simulink model "HvacActrPosnReqFrnt"  (R2022b)
settings: Solver=FixedStepDiscrete  StartTime=0.0  StopTime=10.0  FixedStep=0.2  RelTol=1e-3
(part 1 of 2)
settings Model: Creator=blue.ge  LastModifiedBy=blue.ge
settings DataIOCC: SaveFormat=StructureWithTime
settings OptimizationCC: DefaultParameterBehavior=Inlined  EnhancedBackFolding=on  PassReuseOutputArgsAs=Individual arguments  ZeroExternalMemoryAtStartup=off  ZeroInternalMemoryAtStartup=off  OptimizeBlockOrder=speed  DifferentSizesBufferReuse=on  OptimizationCustomize=off  EfficientTunableParamExpr=on
settings DebuggingCC: ParameterTunabilityLossMsg=error
settings ModelReferenceCC: ModelReferenceNumInstancesAllowed=Single
settings SFSimCC: MATLABDynamicMemAlloc=off
settings RTWCC: Description=AUTOSAR  SystemTargetFile=autosar.tlc  GenCodeOnly=on  TemplateMakefile=ert_default_tmf
settings CodeAppCC: ForceParamTrailComments=on  IgnoreCustomStorageClasses=off  OperatorAnnotations=on  SimulinkDataObjDesc=on  SFDataObjDesc=on  InsertBlockDesc=on
settings STFCustomTargetCC: TargetLangStandard=C99 (ISO)  MultiwordLength=256  ParMdlRefBuildCompliant=on  CompOptLevelCompliant=on  IncludeMdlTerminateFcn=off  CombineOutputUpdateFcns=on  SuppressErrorStatus=on  ERTCustomFileBanners=on  SupportNonFinite=off  SupportComplex=off  SupportContinuousTime=off  SupportNonInlinedSFcns=off  RemoveResetFunc=on  MATLABClassNameForMDSCustomization=Simulink.SoftwareTarget.ERTCustomization  AutosarCompliant=on  UseToolchainInfoCompliant=on  LookupTableObjectStructAxisOrder=2,1,3,4,...  LUTObjectStructOrderEvenSpacing=Size,Table,Breakpoints  SystemTargetFile=autosar.tlc
settings ERTTargetCC: TargetLangStandard=C99 (ISO)  ConcurrentExecutionCompliant=off  IncludeMdlTerminateFcn=off  SuppressErrorStatus=on  SupportNonFinite=off  SupportComplex=off  ModelStepFunctionPrototypeControlCompliant=off  CPPClassGenCompliant=off  AutosarCompliant=on  LookupTableObjectStructAxisOrder=2,1,3,4,...  LUTObjectStructOrderEvenSpacing=Size,Table,Breakpoints  InlinedParameterPlacement=NonHierarchical  ERTCustomFileTemplate=example_file_process.tlc

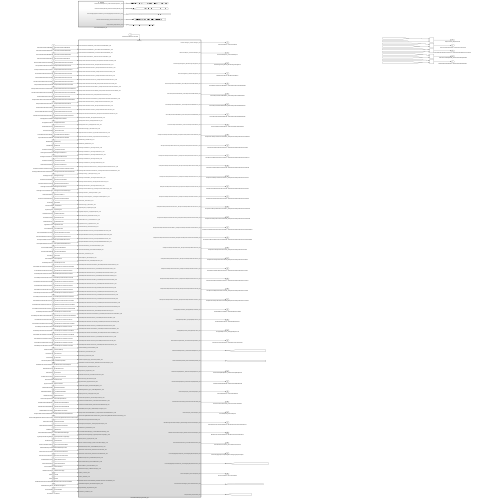
[thumbnail: Simulink's stored preview of the top-level diagram]
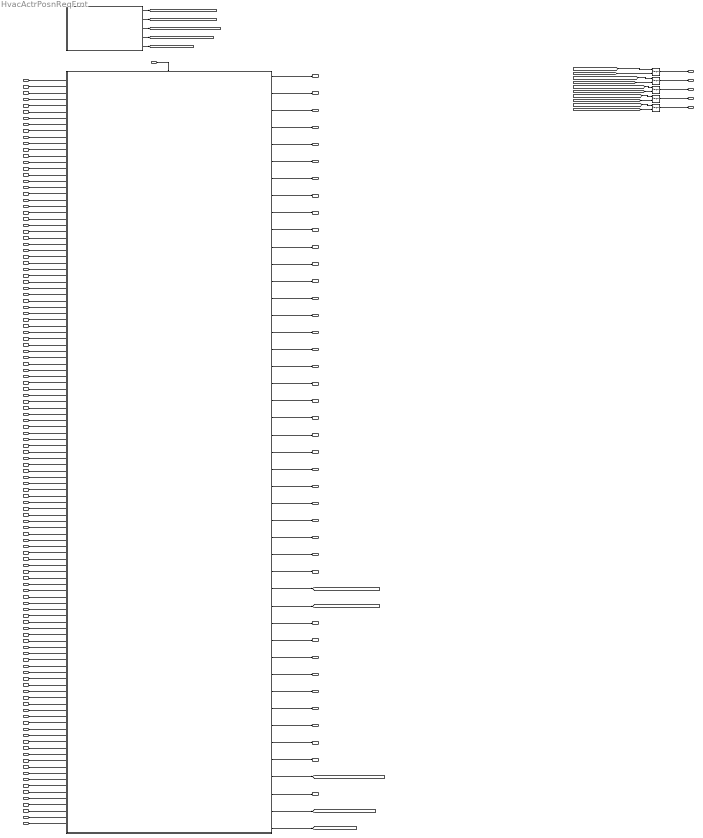
== system HvacActrPosnReqFrnt ==
  HvacActrPosnReqFrnt_Runnable : Inport  SampleTime=0.2  OutputFunctionCall=on
  ActrFrntStsForRowFirstModDistbn_ActrFrntStsForRowFirstModDistbn : Inport  Port=2  OutDataTypeStr=Enum: HvacActrSts  PortDimensions=1
  ActrFrntStsForRowSecModDistbnLe_ActrFrntStsForRowSecModDistbnLe : Inport  Port=3  OutDataTypeStr=Enum: HvacActrSts  PortDimensions=1
  ActrFrntStsForRowSecModDistbnRi_ActrFrntStsForRowSecModDistbnRi : Inport  Port=4  OutDataTypeStr=Enum: HvacActrSts  PortDimensions=1
  ActrPosnFrntActForOsaAndRecirc_ActrPosnFrntActForOsaAndRecirc : Inport  Port=5  OutMin=0  OutMax=100  OutDataTypeStr=Perc9  PortDimensions=1
  AirFlapFrntStsForRowFirstForActrDefrst_AirFlapFrntStsForRowFirstForActrDefrst : Inport  Port=6  OutDataTypeStr=Enum: HvacActrSts  PortDimensions=1
  AirFlapFrntStsForRowFirstForActrFlr_AirFlapFrntStsForRowFirstForActrFlr : Inport  Port=7  OutDataTypeStr=Enum: HvacActrSts  PortDimensions=1
  AirFlapFrntStsForRowFirstForActrFlrLe_AirFlapFrntStsForRowFirstForActrFlrLe : Inport  Port=8  OutDataTypeStr=Enum: HvacActrSts  PortDimensions=1
  AirFlapFrntStsForRowFirstForActrFlrRi_AirFlapFrntStsForRowFirstForActrFlrRi : Inport  Port=9  OutDataTypeStr=Enum: HvacActrSts  PortDimensions=1
  AirFlapFrntStsForRowFirstForActrVent_AirFlapFrntStsForRowFirstForActrVent : Inport  Port=10  OutDataTypeStr=Enum: HvacActrSts  PortDimensions=1
  AirFlapFrntStsForRowFirstForActrVentLe_AirFlapFrntStsForRowFirstForActrVentLe : Inport  Port=11  OutDataTypeStr=Enum: HvacActrSts  PortDimensions=1
  AirFlapFrntStsForRowFirstForActrVentRi_AirFlapFrntStsForRowFirstForActrVentRi : Inport  Port=12  OutDataTypeStr=Enum: HvacActrSts  PortDimensions=1
  AirFlapFrntStsForRowFirstForActrVentSideLe_AirFlapFrntStsForRowFirstForActrVentSideLe : Inport  Port=13  OutDataTypeStr=Enum: HvacActrSts  PortDimensions=1
  AirFlapFrntStsForRowFirstForActrVentSideRi_AirFlapFrntStsForRowFirstForActrVentSideRi : Inport  Port=14  OutDataTypeStr=Enum: HvacActrSts  PortDimensions=1
  AirFlapFrntStsForRowFirstForOsa_AirFlapFrntStsForRowFirstForOsa : Inport  Port=15  OutDataTypeStr=Enum: HvacActrSts  PortDimensions=1
  AirFlapFrntStsForRowFirstForOsaAndRecirc_AirFlapFrntStsForRowFirstForOsaAndRecirc : Inport  Port=16  OutDataTypeStr=Enum: HvacActrSts  PortDimensions=1
  AirFlapFrntStsForRowFirstForRecirc_AirFlapFrntStsForRowFirstForRecirc : Inport  Port=17  OutDataTypeStr=Enum: HvacActrSts  PortDimensions=1
  AirFlapFrntStsForRowSecForActrFlr_AirFlapFrntStsForRowSecForActrFlr : Inport  Port=18  OutDataTypeStr=Enum: HvacActrSts  PortDimensions=1
  AirFlapFrntStsForRowSecForActrVent_AirFlapFrntStsForRowSecForActrVent : Inport  Port=19  OutDataTypeStr=Enum: HvacActrSts  PortDimensions=1
  AirFlapPosnFrntActForRowFirstForRecirc_AirFlapPosnFrntActForRowFirstForRecirc : Inport  Port=20  OutMin=0  OutMax=100  OutDataTypeStr=Perc9  PortDimensions=1
  AirFlapSpdActForAirDistbn_AirFlapSpdActForAirDistbn : Inport  Port=21  OutDataTypeStr=Enum: HvacActrSpd  PortDimensions=1
  AirFlapSpdActForRecirc_AirFlapSpdActForRecirc : Inport  Port=22  OutDataTypeStr=Enum: HvacActrSpd  PortDimensions=1
  AirFlapSpdActForTFrnt_AirFlapSpdActForTFrnt : Inport  Port=23  OutDataTypeStr=Enum: HvacActrSpd  PortDimensions=1
  AmbTFildForClimaNgcc_AmbTFildForClima : Inport  Port=24  OutDataTypeStr=T30  PortDimensions=1
  AmprFanBoostVentnCnsReIntLe_AmprFanBoostVentnCnsReIntLe : Inport  Port=25  OutDataTypeStr=AmprFanBoostVentnCnsRe  PortDimensions=1
  AmprFanBoostVentnCnsReIntRi_AmprFanBoostVentnCnsReIntRi : Inport  Port=26  OutDataTypeStr=AmprFanBoostVentnCnsRe  PortDimensions=1
  BlwAftRunReq_BlwAftRunReq : Inport  Port=27  OutDataTypeStr=Boolean  PortDimensions=1
  BlwAftRunSts_BlwAftRunSts : Inport  Port=28  OutDataTypeStr=Boolean  PortDimensions=1
  ClimaCfgForActrModVrnt1_ClimaCfgForActrInd1 : Inport  Port=29  OutDataTypeStr=Enum: ClimaCfgForActrInd1  PortDimensions=1
  ClimaCfgForActrModVrnt1_ClimaCfgForActrModVrnt1 : Inport  Port=30  OutDataTypeStr=Enum: ClimaCfgForActrModVrnt1  PortDimensions=1
  ClimaCfgForActrModVrnt1_ClimaCfgForActrModVrntCen : Inport  Port=31  OutDataTypeStr=Enum: ClimaCfgForActrModVrntCen  PortDimensions=1
  ClimaCfgForActrModVrnt2_ClimaCfgForActrInd2 : Inport  Port=32  OutDataTypeStr=Enum: ClimaCfgForActrInd2  PortDimensions=1
  ClimaCfgForActrModVrnt2_ClimaCfgForActrModVrnt2 : Inport  Port=33  OutDataTypeStr=Enum: ClimaCfgForActrModVrnt2  PortDimensions=1
  ClimaCfgForActrRecircAndOsaCmnPrsnt1_ClimaCfgForActrRecircAndOsaCmnPrsnt1 : Inport  Port=34  OutDataTypeStr=Enum: ClimaCfgForActrRecircAndOsaCmnPrsnt1  PortDimensions=1
  ClimaCfgForBlowrInRowSecVentSidePrsnt1_ClimaCfgForBlowrInRowSecVentSidePrsnt1 : Inport  Port=35  OutDataTypeStr=Boolean  PortDimensions=1
  ClimaCfgForCarTyp1_ClimaCfgForCarTyp1 : Inport  Port=36  OutDataTypeStr=Enum: ClimaCfgForCarTyp1  PortDimensions=1
  ClimaCfgForClimaCtrlMan1_ClimaCfgForClimaCtrlMan1 : Inport  Port=37  OutDataTypeStr=Boolean  PortDimensions=1
  ClimaCfgForExtVentnClimaCtrl_ClimaCfgForExtVentnClimaCtrl : Inport  Port=38  OutDataTypeStr=Enum: CarCfgForExtVentnClimaCtrl  PortDimensions=1
  ClimaCfgForFanPotiCtrld1_ClimaCfgForFanPotiCtrld1 : Inport  Port=39  OutDataTypeStr=Boolean  PortDimensions=1
  ClimaCfgForHvacFrontBlowerType_ClimaCfgForHvacFrontBlowerType : Inport  Port=40  OutDataTypeStr=Enum: ClimaCfgForHvacFrontBlowerType  PortDimensions=1
  ClimaCfgForVehElec1_ClimaCfgForVehElec1 : Inport  Port=41  OutDataTypeStr=Boolean  PortDimensions=1
  ClimaCfgForVrntOfVehSysElec1_ClimaCfgForVrntOfVehSysElec1 : Inport  Port=42  OutDataTypeStr=Enum: ClimaCenCfgForVrntOfVehSysElec1  PortDimensions=1
  ClimaClsDwn_ClimaClsDwn : Inport  Port=43  OutDataTypeStr=Boolean  PortDimensions=1
  ClimaCtrlActvNgcc_ClimaCtrlActv : Inport  Port=44  OutDataTypeStr=Boolean  PortDimensions=1
  CmptmtClngActv_CmptmtClngActv : Inport  Port=45  OutDataTypeStr=Enum: CmptmtClngActv  PortDimensions=1
  CoolgReqForDispActv_CoolgReqForDispActv : Inport  Port=46  OutDataTypeStr=Enum: CoolgReqForDisp  PortDimensions=1
  ExtVentStsFrmClima_ExtVentStsFrmClima : Inport  Port=47  OutDataTypeStr=Enum: OnOff1  PortDimensions=1
  FlowRednAtPwrLvlLo_FlowRednAtPwrLvlLo : Inport  Port=48  OutDataTypeStr=Boolean  PortDimensions=1
  FogFlashPrevnActv_FogFlashPrevnActv : Inport  Port=49  OutDataTypeStr=Boolean  PortDimensions=1
  FrntHvacBlowerSts_FrntHvacBlowerSts : Inport  Port=50  OutDataTypeStr=Enum: HvacFanSts  PortDimensions=1
  HmiCmptmtAirDistbnFrntForClima_HmiCmptmtAirDistbnFrntForClima : Inport  Port=51  OutDataTypeStr=Enum: HmiCmptmtAirDistbnFrnt  PortDimensions=1
  HmiCmptmtAirDistbnFrntForClima_HmiCmptmtAirDistbnFrntRiForClima : Inport  Port=52  OutDataTypeStr=Enum: HmiCmptmtAirDistbnFrnt  PortDimensions=1
  HmiCmptmtAirDistbnFrntForClima_HmiCmptmtAirDistbnReForClima : Inport  Port=53  OutDataTypeStr=Enum: HmiCmptmtAirDistbnFrnt  PortDimensions=1
  HmiCmptmtAirDistbnFrntForClima_HmiCmptmtAirDistbnReRiForClima : Inport  Port=54  OutDataTypeStr=Enum: HmiCmptmtAirDistbnFrnt  PortDimensions=1
  HvacCtrlrFanBoostReqLe_HvacCtrlrFanBoostReqLe : Inport  Port=55  OutDataTypeStr=Perc30  PortDimensions=1
  HvacCtrlrFanBoostReqRi_HvacCtrlrFanBoostReqRi : Inport  Port=56  OutDataTypeStr=Perc30  PortDimensions=1
  HvacFanIFrnt_HvacFanIFrnt : Inport  Port=57  OutDataTypeStr=HvacFanI  PortDimensions=1
  HvacFanSpdFrnt_HvacFanSpdFrnt : Inport  Port=58  OutDataTypeStr=HvacFanSpd  PortDimensions=1
  HvacFildReqFrntForFan_HvacFildReqFrntForFan : Inport  Port=59  OutDataTypeStr=HvacFanSpd  PortDimensions=1
  HvacFildReqFrntForRowFirstForActrDefrst_HvacFildReqFrntForRowFirstForActrDefrst : Inport  Port=60  OutDataTypeStr=Perc30  PortDimensions=1
  HvacFildReqFrntForRowFirstForActrFlr_HvacFildReqFrntForRowFirstForActrFlr : Inport  Port=61  OutDataTypeStr=Perc30  PortDimensions=1
  HvacFildReqFrntForRowFirstForActrFlr_HvacFildReqFrntForRowFirstForActrFlrLe : Inport  Port=62  OutDataTypeStr=Perc30  PortDimensions=1
  HvacFildReqFrntForRowFirstForActrFlr_HvacFildReqFrntForRowFirstForActrFlrRi : Inport  Port=63  OutDataTypeStr=Perc30  PortDimensions=1
  HvacFildReqFrntForRowFirstForActrMod_HvacFildReqFrntForRowFirstForActrMod : Inport  Port=64  OutDataTypeStr=Perc30  PortDimensions=1
  HvacFildReqFrntForRowFirstForActrTLe_HvacFildReqFrntForRowFirstForActrTLe : Inport  Port=65  OutDataTypeStr=Perc30  PortDimensions=1
  HvacFildReqFrntForRowFirstForActrTRi_HvacFildReqFrntForRowFirstForActrTRi : Inport  Port=66  OutDataTypeStr=Perc30  PortDimensions=1
  HvacFildReqFrntForRowFirstForActrVent_HvacFildReqFrntForRowFirstForActrVent : Inport  Port=67  OutDataTypeStr=Perc30  PortDimensions=1
  HvacFildReqFrntForRowFirstForActrVent_HvacFildReqFrntForRowFirstForActrVentLe : Inport  Port=68  OutDataTypeStr=Perc30  PortDimensions=1
  HvacFildReqFrntForRowFirstForActrVent_HvacFildReqFrntForRowFirstForActrVentRi : Inport  Port=69  OutDataTypeStr=Perc30  PortDimensions=1
  HvacFildReqFrntForRowFirstForActrVent_HvacFildReqFrntForRowFirstForActrVentSideLe : Inport  Port=70  OutDataTypeStr=Perc30  PortDimensions=1
  HvacFildReqFrntForRowFirstForActrVent_HvacFildReqFrntForRowFirstForActrVentSideRi : Inport  Port=71  OutDataTypeStr=Perc30  PortDimensions=1
  HvacFildReqFrntForRowFirstForOsa_HvacFildReqFrntForRowFirstForOsa : Inport  Port=72  OutDataTypeStr=Perc30  PortDimensions=1
  HvacFildReqFrntForRowFirstForOsaAndRecirc_HvacFildReqFrntForRowFirstForOsaAndRecirc : Inport  Port=73  OutDataTypeStr=Perc30  PortDimensions=1
  HvacFildReqFrntForRowFirstForRecirc_HvacFildReqFrntForRowFirstForRecirc : Inport  Port=74  OutDataTypeStr=Perc30  PortDimensions=1
  HvacFildReqFrntForRowFirstLeForActrMod_HvacFildReqFrntForRowFirstLeForActrMod : Inport  Port=75  OutDataTypeStr=Perc30  PortDimensions=1
  HvacFildReqFrntForRowSecForActrFlr_HvacFildReqFrntForRowSecForActrFlr : Inport  Port=76  OutDataTypeStr=Perc30  PortDimensions=1
  HvacFildReqFrntForRowSecForActrModLe_HvacFildReqFrntForRowSecForActrModLe : Inport  Port=77  OutDataTypeStr=Perc30  PortDimensions=1
  HvacFildReqFrntForRowSecForActrModRi_HvacFildReqFrntForRowSecForActrModRi : Inport  Port=78  OutDataTypeStr=Perc30  PortDimensions=1
  HvacFildReqFrntForRowSecForActrTLe_HvacFildReqFrntForRowSecForActrTLe : Inport  Port=79  OutDataTypeStr=Perc30  PortDimensions=1
  HvacFildReqFrntForRowSecForActrTRi_HvacFildReqFrntForRowSecForActrTRi : Inport  Port=80  OutDataTypeStr=Perc30  PortDimensions=1
  HvacFildReqFrntForRowSecForActrVent_HvacFildReqFrntForRowSecForActrVent : Inport  Port=81  OutDataTypeStr=Perc30  PortDimensions=1
  HvacRecircRatReq_HvacRecircRatReq : Inport  Port=82  OutDataTypeStr=Perc30  PortDimensions=1
  HvacTarFlowTot_HvacTarFlowTot : Inport  Port=83  OutDataTypeStr=AirFlow30  PortDimensions=1
  IntCo2ActvSts_IntCo2ActvSts : Inport  Port=84  OutDataTypeStr=Enum: IntCo2Sts  PortDimensions=1
  InteCleanUnpleSmell_Ngcc_InteCleanUnpleSmell : Inport  Port=85  OutDataTypeStr=Enum: OnOff1  PortDimensions=1
  MemReadForHvacActrPosnReqFrnt_MemReadForHvacActrPosnReqFrnt : Inport  Port=86  OutDataTypeStr=Bus: MemRecForHvacActrPosnReqFrnt  PortDimensions=1  BusOutputAsStruct=on
  MemReqStsForHvac_MemReqStsForHvac : Inport  Port=87  OutDataTypeStr=Enum: MemReqSts  PortDimensions=1
  PrkgClimaActv_PrkgClimaActv : Inport  Port=88  OutDataTypeStr=Enum: PrkgClimaActv  PortDimensions=1
  PwrAllwdForHvacFanFrnt_PwrAllwdForHvacFanFrnt : Inport  Port=89  OutDataTypeStr=Pwr33  PortDimensions=1
  RemVentActvSts_RemVentActvSts : Inport  Port=90  OutDataTypeStr=Enum: OnOff1  PortDimensions=1
  SplyUForClimaCtrl_SplyUForClimaCtrl : Inport  Port=91  OutDataTypeStr=BattU  PortDimensions=1
  TWinRfClsdPopUpReq_TWinRfClsdPopUpReq : Inport  Port=92  OutDataTypeStr=Enum: OnOff1  PortDimensions=1
  VehModMngtGlbSafe1_Ngcc_VehModMngtGlbSafe1 : Inport  Port=93  OutDataTypeStr=Bus: VehModMngtGlbSafe1  PortDimensions=1  BusOutputAsStruct=on
  VehSpdLgtForClima_VehSpdLgtForClima : Inport  Port=94  OutDataTypeStr=Bus: VehSpdLgtForClima  PortDimensions=1  BusOutputAsStruct=on
  VentnForWinOpenGlbActv_VentnForWinOpenGlbActv : Inport  Port=95  OutDataTypeStr=Boolean  PortDimensions=1
  VoltFanBoostVentnMeasReIntLe_VoltFanBoostVentnMeasReIntLe : Inport  Port=96  OutDataTypeStr=VoltFanBoostVentnMeasRe  PortDimensions=1
  VoltFanBoostVentnMeasReIntRi_VoltFanBoostVentnMeasReIntRi : Inport  Port=97  OutDataTypeStr=VoltFanBoostVentnMeasRe  PortDimensions=1
  WinRfClsdReqForCoolgDwn_WinRfClsdReqForCoolgDwn : Inport  Port=98  OutDataTypeStr=Enum: Warn2  PortDimensions=1
  AirFlapFrntStsForRowFirstModDistbnLe_AirFlapFrntStsForRowFirstModDistbnLe : Inport  Port=99  OutDataTypeStr=Enum: HvacActrSts  PortDimensions=1
  AirFlowLimDurgMidAmbtForNVHForHVACFrntFlag_AirFlowLimDurgMidAmbtForNVHForHVACFrntFlag : Inport  Port=100  OutDataTypeStr=Boolean  PortDimensions=1
  AmbTFildForClimaQf_AmbTFildForClimaQf : Inport  Port=101  OutDataTypeStr=Enum: GenQf1  PortDimensions=1
  ClimaCfgReqVersionReplace_ClimaCfgReqVersionReplace : Inport  Port=102  OutDataTypeStr=UInt8  PortDimensions=1
  FrntBlwWrkLmt_FrntBlwWrkLmt : Inport  Port=103  OutDataTypeStr=UInt8  PortDimensions=1
  FrntClimaManModCmftStsNgcc_FrntClimaManModCmftStsNgcc : Inport  Port=104  OutDataTypeStr=Enum: ClimaManModCmftSts  PortDimensions=1
  HighTempCleanRunningStsNgcc_HighTempCleanRunningStsNgcc : Inport  Port=105  OutDataTypeStr=Enum: OnOffNoReq  PortDimensions=1
  HighTempClnActv_HighTempClnActv : Inport  Port=106  OutDataTypeStr=UInt8  PortDimensions=1
  HmiCarLockACRecircSetNgcc_HmiCarLockACRecircSetNgcc : Inport  Port=107  OutMin=0  OutMax=3  OutDataTypeStr=UInt2  PortDimensions=1
  HmiDefrstMaxReqForClima_HmiDefrstMaxReqForClima : Inport  Port=108  OutDataTypeStr=Enum: ActrReq  PortDimensions=1
  HmiHvacFanLvlFrntForClima_HmiHvacFanLvlFrntForClima : Inport  Port=109  OutDataTypeStr=Enum: HmiHvacFanLvl  PortDimensions=1
  HmiHvacFanLvlFrntForClima_HmiHvacFanLvlFrntRiForClima : Inport  Port=110  OutDataTypeStr=Enum: HmiHvacFanLvl  PortDimensions=1
  HmiMaxACReqForClima_HmiMaxACReqForClima : Inport  Port=111  OutDataTypeStr=Enum: OnOff1  PortDimensions=1
  HvacPwm2MosVFbFrnt_HvacPwm2MosVFbFrnt : Inport  Port=112  OutMin=0  OutMax=100  OutDataTypeStr=Perc9  PortDimensions=1
  HvacPwmNFbFrnt_HvacPwmNFbFrnt : Inport  Port=113  OutDataTypeStr=HvacFanSpd  PortDimensions=1
  HvBattLimnIndcnNgcc_HvBattLimnIndcnNgcc : Inport  Port=114  OutDataTypeStr=UInt16  PortDimensions=1
  LdAdVal_CurrAdVal : Inport  Port=115  OutDataTypeStr=Bus: LdAd  PortDimensions=1  BusOutputAsStruct=on
  LdAdVal_ValidAdVal : Inport  Port=116  OutDataTypeStr=Bus: LdAd  PortDimensions=1  BusOutputAsStruct=on
  ManModeForRowFirstLeForModelDial_ManModeForRowFirstLeForModelDial : Inport  Port=117  OutDataTypeStr=Enum: HmiCmptmtAirDistbnFrnt  PortDimensions=1
  MemReadForPrkgRecirc_MemReadForPrkgRecirc : Inport  Port=118  OutDataTypeStr=UInt8  PortDimensions=1
  PrkgClimaOnReq_PrkgClimaOnReq : Inport  Port=119  OutDataTypeStr=Boolean  PortDimensions=1
  PrvntWinFrz_PrvntWinFrz : Inport  Port=120  OutDataTypeStr=Boolean  PortDimensions=1
  FanBoostVentnRampReIntLe_FanBoostVentnRampReIntLe_merge : Merge
  FanBoostVentnRampReIntRi_FanBoostVentnRampReIntRi_merge : Merge
  HvacActrPosnReqFrnt_Init : Subsystem
  HvacActrPosnReqFrnt_Runnable_sys : Subsystem
  PwrCnsDurgEngStopForHvacFanFrnt_PwrCnsDurgEngStopForHvacFanFrnt_merge : Merge
  PwrCnsForHvacFanFrntNgcc_PwrCnsForHvacFanFrnt_merge : Merge
  ReqForActrCal_ReqForActrCal_merge : Merge
  FanBoostVentnRampReIntLe_FanBoostVentnRampReIntLe : Outport  OutDataTypeStr=FanBoostPercPerTiUnit  PortDimensions=1
  FanBoostVentnRampReIntRi_FanBoostVentnRampReIntRi : Outport  Port=2  OutDataTypeStr=FanBoostPercPerTiUnit  PortDimensions=1
  PwrCnsDurgEngStopForHvacFanFrnt_PwrCnsDurgEngStopForHvacFanFrnt : Outport  Port=3  OutDataTypeStr=Pwr33  PortDimensions=1
  PwrCnsForHvacFanFrntNgcc_PwrCnsForHvacFanFrnt : Outport  Port=4  OutDataTypeStr=Pwr33  PortDimensions=1
  ReqForActrCal_ReqForActrCal : Outport  Port=5  OutDataTypeStr=Boolean  PortDimensions=1
  HvacFanLvlFbNgcc_HvacFanLvlFbNgcc : Outport  Port=6  OutDataTypeStr=Enum: HmiHvacFanLvl  PortDimensions=1
  HvacFanVoltReqFrnt_HvacFanVoltReqFrnt : Outport  Port=7  OutMin=0  OutMax=100  OutDataTypeStr=Perc9  PortDimensions=1
  MemSaveFlgForPrkgRecirc_MemSaveFlgForPrkgRecirc : Outport  Port=8  OutDataTypeStr=Boolean  PortDimensions=1
  MemWrForPrkgRecirc_MemWrForPrkgRecirc : Outport  Port=9  OutDataTypeStr=UInt8  PortDimensions=1
  ActrFrntReqForRowFirstLeModDistbn_ActrFrntReqForRowFirstLeModDistbn : Outport  Port=10  OutMin=0  OutMax=100  OutDataTypeStr=Perc9  PortDimensions=1
  ActrFrntReqForRowFirstModDistbn_ActrFrntReqForRowFirstModDistbn : Outport  Port=11  OutMin=0  OutMax=100  OutDataTypeStr=Perc9  PortDimensions=1
  ActrFrntReqForRowSecModDistbnLe_ActrFrntReqForRowSecModDistbnLe : Outport  Port=12  OutMin=0  OutMax=100  OutDataTypeStr=Perc9  PortDimensions=1
  ActrFrntReqForRowSecModDistbnRi_ActrFrntReqForRowSecModDistbnRi : Outport  Port=13  OutMin=0  OutMax=100  OutDataTypeStr=Perc9  PortDimensions=1
  ActrPosnFrnReqForOsaAndRecirc_ActrPosnFrnReqForOsaAndRecirc : Outport  Port=14  OutMin=0  OutMax=100  OutDataTypeStr=Perc9  PortDimensions=1
  AirFlapPosnFrntReqForRowFirstForActrDefrst_AirFlapPosnFrntReqForRowFirstForActrDefrst : Outport  Port=15  OutMin=0  OutMax=100  OutDataTypeStr=Perc9  PortDimensions=1
  AirFlapPosnFrntReqForRowFirstForActrFlr_AirFlapPosnFrntReqForRowFirstForActrFlr : Outport  Port=16  OutMin=0  OutMax=100  OutDataTypeStr=Perc9  PortDimensions=1
  AirFlapPosnFrntReqForRowFirstForActrFlrLe_AirFlapPosnFrntReqForRowFirstForActrFlrLe : Outport  Port=17  OutMin=0  OutMax=100  OutDataTypeStr=Perc9  PortDimensions=1
  AirFlapPosnFrntReqForRowFirstForActrFlrRi_AirFlapPosnFrntReqForRowFirstForActrFlrRi : Outport  Port=18  OutMin=0  OutMax=100  OutDataTypeStr=Perc9  PortDimensions=1
  AirFlapPosnFrntReqForRowFirstForActrTLe_AirFlapPosnFrntReqForRowFirstForActrTLe : Outport  Port=19  OutMin=0  OutMax=100  OutDataTypeStr=Perc9  PortDimensions=1
  AirFlapPosnFrntReqForRowFirstForActrTRi_AirFlapPosnFrntReqForRowFirstForActrTRi : Outport  Port=20  OutMin=0  OutMax=100  OutDataTypeStr=Perc9  PortDimensions=1
  AirFlapPosnFrntReqForRowFirstForActrVent_AirFlapPosnFrntReqForRowFirstForActrVent : Outport  Port=21  OutMin=0  OutMax=100  OutDataTypeStr=Perc9  PortDimensions=1
  AirFlapPosnFrntReqForRowFirstForActrVentLe_AirFlapPosnFrntReqForRowFirstForActrVentLe : Outport  Port=22  OutMin=0  OutMax=100  OutDataTypeStr=Perc9  PortDimensions=1
  AirFlapPosnFrntReqForRowFirstForActrVentRi_AirFlapPosnFrntReqForRowFirstForActrVentRi : Outport  Port=23  OutMin=0  OutMax=100  OutDataTypeStr=Perc9  PortDimensions=1
  AirFlapPosnFrntReqForRowFirstForActrVentSideLe_AirFlapPosnFrntReqForRowFirstForActrVentSideLe : Outport  Port=24  OutMin=0  OutMax=100  OutDataTypeStr=Perc9  PortDimensions=1
  AirFlapPosnFrntReqForRowFirstForActrVentSideRi_AirFlapPosnFrntReqForRowFirstForActrVentSideRi : Outport  Port=25  OutMin=0  OutMax=100  OutDataTypeStr=Perc9  PortDimensions=1
  AirFlapPosnFrntReqForRowFirstForOsa_AirFlapPosnFrntReqForRowFirstForOsa : Outport  Port=26  OutMin=0  OutMax=100  OutDataTypeStr=Perc9  PortDimensions=1
  AirFlapPosnFrntReqForRowFirstForRecirc_AirFlapPosnFrntReqForRowFirstForRecirc : Outport  Port=27  OutMin=0  OutMax=100  OutDataTypeStr=Perc9  PortDimensions=1
  AirFlapPosnFrntReqForRowSecForActrFlr_AirFlapPosnFrntReqForRowSecForActrFlr : Outport  Port=28  OutMin=0  OutMax=100  OutDataTypeStr=Perc9  PortDimensions=1
  AirFlapPosnFrntReqForRowSecForActrTLe_AirFlapPosnFrntReqForRowSecForActrTLe : Outport  Port=29  OutMin=0  OutMax=100  OutDataTypeStr=Perc9  PortDimensions=1
  AirFlapPosnFrntReqForRowSecForActrTRi_AirFlapPosnFrntReqForRowSecForActrTRi : Outport  Port=30  OutMin=0  OutMax=100  OutDataTypeStr=Perc9  PortDimensions=1
  AirFlapPosnFrntReqForRowSecForActrVent_AirFlapPosnFrntReqForRowSecForActrVent : Outport  Port=31  OutMin=0  OutMax=100  OutDataTypeStr=Perc9  PortDimensions=1
  AirFlapSpdReqForAirDistbn_AirFlapSpdReqForAirDistbn : Outport  Port=32  OutDataTypeStr=Enum: HvacActrSpd  PortDimensions=1
  AirFlapSpdReqForRecirc_AirFlapSpdReqForRecirc : Outport  Port=33  OutDataTypeStr=Enum: HvacActrSpd  PortDimensions=1
  AirFlapSpdReqForTFrnt_AirFlapSpdReqForTFrnt : Outport  Port=34  OutDataTypeStr=Enum: HvacActrSpd  PortDimensions=1
  ClimaCtrlVFCClnCmptmtClima_ClimaCtrlVFCClnCmptmtClima : Outport  Port=35  OutDataTypeStr=Enum: OnOff1  PortDimensions=1
  FanBoostVentnSpReqReIntLe_FanBoostVentnSpReqReIntLe : Outport  Port=36  OutDataTypeStr=FanBoostPercHalfResl  PortDimensions=1
  FanBoostVentnSpReqReIntRi_FanBoostVentnSpReqReIntRi : Outport  Port=37  OutDataTypeStr=FanBoostPercHalfResl  PortDimensions=1
  HvacFanDutyReqFrnt_HvacFanDutyReqFrnt : Outport  Port=38  OutMin=0  OutMax=100  OutDataTypeStr=Perc9  PortDimensions=1
  HvacFanFrntLimForPwrAllwd_HvacFanFrntLimForPwrAllwd : Outport  Port=39  OutDataTypeStr=Enum: HvacFanFrntLimForPwrAllwd  PortDimensions=1
  HvacFanReqFrnt_HvacFanReqFrnt : Outport  Port=40  OutDataTypeStr=HvacFanSpd  PortDimensions=1
  MemSaveFlgForHvacActrPosnReqFrnt_MemSaveFlgForHvacActrPosnReqFrnt : Outport  Port=41  OutDataTypeStr=Boolean  PortDimensions=1
  MemWrForHvacActrPosnReqFrnt_MemWrForHvacActrPosnReqFrnt : Outport  Port=42  OutDataTypeStr=Bus: MemRecForHvacActrPosnReqFrnt  PortDimensions=1  BusOutputAsStruct=on
  PwrCnsDesForHvacFanFrnt_PwrCnsDesForHvacFanFrnt : Outport  Port=43  OutDataTypeStr=Pwr33  PortDimensions=1
  PwrCnsDurgEngStopForFlapActr_PwrCnsDurgEngStopForFlapActr : Outport  Port=44  OutDataTypeStr=Pwr33  PortDimensions=1
  PwrCnsForFlapActr_PwrCnsForFlapActr : Outport  Port=45  OutDataTypeStr=Pwr33  PortDimensions=1
  wires:
  ActrFrntStsForRowFirstModDistbn_ActrFrntStsForRowFirstModDistbn/1 -> HvacActrPosnReqFrnt_Runnable_sys/1(ActrFrntStsForRowFirstModDistbn_ActrFrntStsForRowFirstModDistbn_read)
  ActrFrntStsForRowSecModDistbnLe_ActrFrntStsForRowSecModDistbnLe/1 -> HvacActrPosnReqFrnt_Runnable_sys/2(ActrFrntStsForRowSecModDistbnLe_ActrFrntStsForRowSecModDistbnLe_read)
  ActrFrntStsForRowSecModDistbnRi_ActrFrntStsForRowSecModDistbnRi/1 -> HvacActrPosnReqFrnt_Runnable_sys/3(ActrFrntStsForRowSecModDistbnRi_ActrFrntStsForRowSecModDistbnRi_read)
  ActrPosnFrntActForOsaAndRecirc_ActrPosnFrntActForOsaAndRecirc/1 -> HvacActrPosnReqFrnt_Runnable_sys/4(ActrPosnFrntActForOsaAndRecirc_ActrPosnFrntActForOsaAndRecirc_read)
  AirFlapFrntStsForRowFirstForActrDefrst_AirFlapFrntStsForRowFirstForActrDefrst/1 -> HvacActrPosnReqFrnt_Runnable_sys/5(AirFlapFrntStsForRowFirstForActrDefrst_AirFlapFrntStsForRowFirstForActrDefrst_read)
  AirFlapFrntStsForRowFirstForActrFlr_AirFlapFrntStsForRowFirstForActrFlr/1 -> HvacActrPosnReqFrnt_Runnable_sys/6(AirFlapFrntStsForRowFirstForActrFlr_AirFlapFrntStsForRowFirstForActrFlr_read)
  AirFlapFrntStsForRowFirstForActrFlrLe_AirFlapFrntStsForRowFirstForActrFlrLe/1 -> HvacActrPosnReqFrnt_Runnable_sys/7(AirFlapFrntStsForRowFirstForActrFlrLe_AirFlapFrntStsForRowFirstForActrFlrLe_read)
  AirFlapFrntStsForRowFirstForActrFlrRi_AirFlapFrntStsForRowFirstForActrFlrRi/1 -> HvacActrPosnReqFrnt_Runnable_sys/8(AirFlapFrntStsForRowFirstForActrFlrRi_AirFlapFrntStsForRowFirstForActrFlrRi_read)
  AirFlapFrntStsForRowFirstForActrVent_AirFlapFrntStsForRowFirstForActrVent/1 -> HvacActrPosnReqFrnt_Runnable_sys/9(AirFlapFrntStsForRowFirstForActrVent_AirFlapFrntStsForRowFirstForActrVent_read)
  AirFlapFrntStsForRowFirstForActrVentLe_AirFlapFrntStsForRowFirstForActrVentLe/1 -> HvacActrPosnReqFrnt_Runnable_sys/10(AirFlapFrntStsForRowFirstForActrVentLe_AirFlapFrntStsForRowFirstForActrVentLe_read)
  AirFlapFrntStsForRowFirstForActrVentRi_AirFlapFrntStsForRowFirstForActrVentRi/1 -> HvacActrPosnReqFrnt_Runnable_sys/11(AirFlapFrntStsForRowFirstForActrVentRi_AirFlapFrntStsForRowFirstForActrVentRi_read)